simulink model "dds_cwg_32k_fft_only_wb"  (R2018a) (continued)
== system dds_cwg_32k_fft_only_wb == (continued)
      mask init:
      | simple_bram_vacc_init(gcb, ...
      |     'vec_len', vec_len, ...
      |     'arith_type', arith_type, ...
      |     'n_bits', n_bits, ...
      |     'bin_pt', bin_pt);
      mask TypeOptions arith_type: Signed | Unsigned
  simple_bram_vacc3 : Subsystem  Commented=on  AncestorBlock=casper_library_accumulators/simple_bram_vacc  mask(simple_bram_vacc){vec_len=2^13, arith_type=Signed, n_bits=32, bin_pt=7}
      mask prompts: vec_len "Vector length:"; arith_type "Output type:"; n_bits "Bit width (output):"; bin_pt "Binary point (output):"
      mask help: This simple vector accumulator is based on a shift register. It outputs its previous accumulated vector after a new_acc pulse is received.
      mask init:
      | simple_bram_vacc_init(gcb, ...
      |     'vec_len', vec_len, ...
      |     'arith_type', arith_type, ...
      |     'n_bits', n_bits, ...
      |     'bin_pt', bin_pt);
      mask TypeOptions arith_type: Signed | Unsigned
  simple_bram_vacc8 : Subsystem  Commented=on  AncestorBlock=casper_library_accumulators/simple_bram_vacc  mask(simple_bram_vacc){vec_len=2^13, arith_type=Signed, n_bits=32, bin_pt=7}
      mask prompts: vec_len "Vector length:"; arith_type "Output type:"; n_bits "Bit width (output):"; bin_pt "Binary point (output):"
      mask help: This simple vector accumulator is based on a shift register. It outputs its previous accumulated vector after a new_acc pulse is received.
      mask init:
      | simple_bram_vacc_init(gcb, ...
      |     'vec_len', vec_len, ...
      |     'arith_type', arith_type, ...
      |     'n_bits', n_bits, ...
      |     'bin_pt', bin_pt);
      mask TypeOptions arith_type: Signed | Unsigned
  snap_adc : Subsystem  Tag=casper:bitsnap  AncestorBlock=casper_library_scopes/bitfield_snapshot  mask(bitsnap){io_names=[p0_d0 p0_d1 p0_d2 p0_d3 p0_d4 p0_d5 p0_d6 p0_d7], io_widths=10, io_bps=9, io_types=1, show_format=on, snap_storage=bram, snap_dram_dimm=2, snap_dram_clock=250, snap_nsamples=10, snap_data_width=128, snap_offset=off, snap_circap=off, snap_value=off, snap_ext_arm=off, snap_ext_circ=off, snap_provide_outputs=on, snap_use_dsp48=on, snap_delay=2, extra_names=timestamp, extra_widths=32, extra_bps=0, extra_types=0}
      mask prompts: io_names "Names [msb...lsb]"; io_widths "Bitwidths [msb...lsb]"; io_bps "Binary pts [msb...lsb]"; io_types "Data types [msb...lsb], ufix=0, fix=1, bool=2"; show_format "Print format string?"; snap_storage "Storage medium"; snap_dram_dimm "DRAM dimm"; snap_dram_clock "DRAM clock rate"; snap_nsamples "Number of Samples (2^?)"; snap_data_width "Data width"; snap_offset "Start delay support"; snap_circap "Circular capture support"; snap_value "Extra value capture support"; snap_ext_arm "External arm support"; snap_ext_circ "External circular capture support"; snap_provide_outputs "Provide outputs"; snap_use_dsp48 "Use DSP48s to implement counters"; snap_delay "Pre-snapshot delay"; extra_names "Names [msb...lsb]"; extra_widths "Widths [msb...lsb]"; extra_bps "Binary pts [msb...lsb]"; extra_types "Data types [msb...lsb], ufix=0, fix=1, bool=2"
      mask help:
      | A wrapped snapshot block. Allows specification of inputs to a snapblock word so the toolflow can automatically set up the scope.
      | See documentation for snapshot for explanation of parameters.
      mask init:
      | bitsnap_init(gcb);
      settings: AttributesFormatString=|
      | snap(8):f10.9,f10.9,f10.9,f10.9,f10.9,f10.9,f10.9,f10.9 = 80 bits\n128 wide, 1024 deep
      | debugID: 20968299
      mask TypeOptions snap_storage: bram | dram
      mask Callback snap_storage: bitsnap_callback();
      mask TypeOptions snap_dram_dimm: 1 | 2 | 3 | 4
      mask TypeOptions snap_dram_clock: 200 | 250 | 266 | 300 | 333
      mask TypeOptions snap_data_width: 8 | 16 | 32 | 64 | 128
      mask Callback snap_value: bitsnap_callback();
      mask Callback snap_ext_arm: bitsnap_callback();
      mask Callback snap_ext_circ: bitsnap_callback();
      mask Callback snap_provide_outputs: bitsnap_callback();
      mask DialogControl ParameterTabVar0 Prompt: Inputs
      mask DialogControl ParameterTabVar01 Prompt: Snapshot
      mask DialogControl ParameterTabVar012 Prompt: Extra Value
  snap_quant0 : Subsystem  Tag=casper:bitsnap  Commented=on  AncestorBlock=casper_library_scopes/bitfield_snapshot  mask(bitsnap){io_names=[real0 imag0 real1 imag1 real2 imag2 real3 imag3], io_widths=8, io_bps=7, io_types=1, show_format=on, snap_storage=bram, snap_dram_dimm=2, snap_dram_clock=250, snap_nsamples=13, snap_data_width=64, snap_offset=on, snap_circap=off, snap_value=off, snap_ext_arm=off, snap_ext_circ=off, snap_provide_outputs=on, snap_use_dsp48=on, snap_delay=0, extra_names=[c d], extra_widths=[30 2], extra_bps=[0 0], extra_types=0}
      mask prompts: io_names "Names [msb...lsb]"; io_widths "Bitwidths [msb...lsb]"; io_bps "Binary pts [msb...lsb]"; io_types "Data types [msb...lsb], ufix=0, fix=1, bool=2"; show_format "Print format string?"; snap_storage "Storage medium"; snap_dram_dimm "DRAM dimm"; snap_dram_clock "DRAM clock rate"; snap_nsamples "Number of Samples (2^?)"; snap_data_width "Data width"; snap_offset "Start delay support"; snap_circap "Circular capture support"; snap_value "Extra value capture support"; snap_ext_arm "External arm support"; snap_ext_circ "External circular capture support"; snap_provide_outputs "Provide outputs"; snap_use_dsp48 "Use DSP48s to implement counters"; snap_delay "Pre-snapshot delay"; extra_names "Names [msb...lsb]"; extra_widths "Widths [msb...lsb]"; extra_bps "Binary pts [msb...lsb]"; extra_types "Data types [msb...lsb], ufix=0, fix=1, bool=2"
      mask help:
      | A wrapped snapshot block. Allows specification of inputs to a snapblock word so the toolflow can automatically set up the scope.
      | See documentation for snapshot for explanation of parameters.
      mask init:
      | bitsnap_init(gcb);
      settings: AttributesFormatString=|
      | snap(8):f8.7,f8.7,f8.7,f8.7,f8.7,f8.7,f8.7,f8.7 = 64 bits\n64 wide, 8192 deep
      | debugID: 82310566
      mask TypeOptions snap_storage: bram | dram
      mask Callback snap_storage: bitsnap_callback();
      mask TypeOptions snap_dram_dimm: 1 | 2 | 3 | 4
      mask TypeOptions snap_dram_clock: 200 | 250 | 266 | 300 | 333
      mask TypeOptions snap_data_width: 8 | 16 | 32 | 64 | 128
      mask Callback snap_value: bitsnap_callback();
      mask Callback snap_ext_arm: bitsnap_callback();
      mask Callback snap_ext_circ: bitsnap_callback();
      mask Callback snap_provide_outputs: bitsnap_callback();
      mask DialogControl ParameterTabVar0 Prompt: Inputs
      mask DialogControl ParameterTabVar01 Prompt: Snapshot
      mask DialogControl ParameterTabVar012 Prompt: Extra Value
  spect_cnt1 : xbsIndex_r4/Counter  Commented=on  cnt_type=Free Running  cnt_to=Inf  operation=Up  start_count=0  cnt_by_val=1  arith_type=Unsigned  n_bits=32  bin_pt=0  load_pin=off  rst=on  en=on  explicit_period=on  period=1  use_behavioral_HDL=off  implementation=Fabric  use_rpm=off  dbl_ovrd=off  block_type=counter
  spect_cnt2 : xbsIndex_r4/Counter  Commented=on  cnt_type=Free Running  cnt_to=Inf  operation=Up  start_count=0  cnt_by_val=1  arith_type=Unsigned  n_bits=32  bin_pt=0  load_pin=off  rst=on  en=on  explicit_period=on  period=1  use_behavioral_HDL=off  implementation=Fabric  use_rpm=off  dbl_ovrd=off  block_type=counter
  spect_cnt3 : xbsIndex_r4/Counter  Commented=on  cnt_type=Free Running  cnt_to=Inf  operation=Up  start_count=0  cnt_by_val=1  arith_type=Unsigned  n_bits=32  bin_pt=0  load_pin=off  rst=on  en=on  explicit_period=on  period=1  use_behavioral_HDL=off  implementation=Fabric  use_rpm=off  dbl_ovrd=off  block_type=counter
  ss_fft : Subsystem  Tag=casper:bitsnap  AncestorBlock=casper_library_scopes/bitfield_snapshot  mask(bitsnap){io_names=[r0 i0 r1 i1 r2 i2 r3 i3], io_widths=[10 10 10 10 10 10 10 10], io_bps=[9 9 9 9 9 9 9 9], io_types=[1 1 1 1 1 1 1 1], show_format=on, snap_storage=bram, snap_dram_dimm=2, snap_dram_clock=250, snap_nsamples=13, snap_data_width=128, snap_offset=off, snap_circap=off, snap_value=off, snap_ext_arm=off, snap_ext_circ=off, snap_provide_outputs=on, snap_use_dsp48=on, snap_delay=2, extra_names=[c d], extra_widths=[30 2], extra_bps=[0 0], extra_types=[0 0]}
      mask prompts: io_names "Names [msb...lsb]"; io_widths "Bitwidths [msb...lsb]"; io_bps "Binary pts [msb...lsb]"; io_types "Data types [msb...lsb], ufix=0, fix=1, bool=2"; show_format "Print format string?"; snap_storage "Storage medium"; snap_dram_dimm "DRAM dimm"; snap_dram_clock "DRAM clock rate"; snap_nsamples "Number of Samples (2^?)"; snap_data_width "Data width"; snap_offset "Start delay support"; snap_circap "Circular capture support"; snap_value "Extra value capture support"; snap_ext_arm "External arm support"; snap_ext_circ "External circular capture support"; snap_provide_outputs "Provide outputs"; snap_use_dsp48 "Use DSP48s to implement counters"; snap_delay "Pre-snapshot delay"; extra_names "Names [msb...lsb]"; extra_widths "Widths [msb...lsb]"; extra_bps "Binary pts [msb...lsb]"; extra_types "Data types [msb...lsb], ufix=0, fix=1, bool=2"
      mask help:
      | A wrapped snapshot block. Allows specification of inputs to a snapblock word so the toolflow can automatically set up the scope.
      | See documentation for snapshot for explanation of parameters.
      mask init:
      | bitsnap_init(gcb);
      settings: AttributesFormatString=|
      | snap(8):f10.9,f10.9,f10.9,f10.9,f10.9,f10.9,f10.9,f10.9 = 80 bits\n128 wide, 8192 deep
      | debugID: 107946561
      mask TypeOptions snap_storage: bram | dram
      mask Callback snap_storage: bitsnap_callback();
      mask TypeOptions snap_dram_dimm: 1 | 2 | 3 | 4
      mask TypeOptions snap_dram_clock: 200 | 250 | 266 | 300 | 333
      mask TypeOptions snap_data_width: 8 | 16 | 32 | 64 | 128
      mask Callback snap_value: bitsnap_callback();
      mask Callback snap_ext_arm: bitsnap_callback();
      mask Callback snap_ext_circ: bitsnap_callback();
      mask Callback snap_provide_outputs: bitsnap_callback();
      mask DialogControl ParameterTabVar0 Prompt: Inputs
      mask DialogControl ParameterTabVar01 Prompt: Snapshot
      mask DialogControl ParameterTabVar012 Prompt: Extra Value
  ss_quant : Subsystem  Tag=casper:bitsnap  Commented=on  AncestorBlock=casper_library_scopes/bitfield_snapshot  mask(bitsnap){io_names=[r0 i0 r1 i1 r2 i2 r3 i3], io_widths=[8 8 8 8 8 8 8 8], io_bps=[7 7 7 7 7 7 7 7], io_types=[1 1 1 1 1 1 1 1], show_format=on, snap_storage=bram, snap_dram_dimm=2, snap_dram_clock=250, snap_nsamples=13, snap_data_width=64, snap_offset=off, snap_circap=off, snap_value=off, snap_ext_arm=off, snap_ext_circ=off, snap_provide_outputs=on, snap_use_dsp48=on, snap_delay=2, extra_names=[c d], extra_widths=[30 2], extra_bps=[0 0], extra_types=[0 0]}
      mask prompts: io_names "Names [msb...lsb]"; io_widths "Bitwidths [msb...lsb]"; io_bps "Binary pts [msb...lsb]"; io_types "Data types [msb...lsb], ufix=0, fix=1, bool=2"; show_format "Print format string?"; snap_storage "Storage medium"; snap_dram_dimm "DRAM dimm"; snap_dram_clock "DRAM clock rate"; snap_nsamples "Number of Samples (2^?)"; snap_data_width "Data width"; snap_offset "Start delay support"; snap_circap "Circular capture support"; snap_value "Extra value capture support"; snap_ext_arm "External arm support"; snap_ext_circ "External circular capture support"; snap_provide_outputs "Provide outputs"; snap_use_dsp48 "Use DSP48s to implement counters"; snap_delay "Pre-snapshot delay"; extra_names "Names [msb...lsb]"; extra_widths "Widths [msb...lsb]"; extra_bps "Binary pts [msb...lsb]"; extra_types "Data types [msb...lsb], ufix=0, fix=1, bool=2"
      mask help:
      | A wrapped snapshot block. Allows specification of inputs to a snapblock word so the toolflow can automatically set up the scope.
      | See documentation for snapshot for explanation of parameters.
      mask init:
      | bitsnap_init(gcb);
      settings: AttributesFormatString=|
      | snap(8):f8.7,f8.7,f8.7,f8.7,f8.7,f8.7,f8.7,f8.7 = 64 bits\n64 wide, 8192 deep
      | debugID: 40187090
      mask TypeOptions snap_storage: bram | dram
      mask Callback snap_storage: bitsnap_callback();
      mask TypeOptions snap_dram_dimm: 1 | 2 | 3 | 4
      mask TypeOptions snap_dram_clock: 200 | 250 | 266 | 300 | 333
      mask TypeOptions snap_data_width: 8 | 16 | 32 | 64 | 128
      mask Callback snap_value: bitsnap_callback();
      mask Callback snap_ext_arm: bitsnap_callback();
      mask Callback snap_ext_circ: bitsnap_callback();
      mask Callback snap_provide_outputs: bitsnap_callback();
      mask DialogControl ParameterTabVar0 Prompt: Inputs
      mask DialogControl ParameterTabVar01 Prompt: Snapshot
      mask DialogControl ParameterTabVar012 Prompt: Extra Value
  ss_vacc0 : Subsystem  Tag=casper:bitsnap  Commented=on  AncestorBlock=casper_library_scopes/bitfield_snapshot  mask(bitsnap){io_names=[data], io_widths=[32], io_bps=[7], io_types=[1], show_format=on, snap_storage=bram, snap_dram_dimm=2, snap_dram_clock=250, snap_nsamples=13, snap_data_width=32, snap_offset=off, snap_circap=off, snap_value=off, snap_ext_arm=off, snap_ext_circ=off, snap_provide_outputs=on, snap_use_dsp48=on, snap_delay=2, extra_names=[c d], extra_widths=[30 2], extra_bps=[0 0], extra_types=[0 0]}
      mask prompts: io_names "Names [msb...lsb]"; io_widths "Bitwidths [msb...lsb]"; io_bps "Binary pts [msb...lsb]"; io_types "Data types [msb...lsb], ufix=0, fix=1, bool=2"; show_format "Print format string?"; snap_storage "Storage medium"; snap_dram_dimm "DRAM dimm"; snap_dram_clock "DRAM clock rate"; snap_nsamples "Number of Samples (2^?)"; snap_data_width "Data width"; snap_offset "Start delay support"; snap_circap "Circular capture support"; snap_value "Extra value capture support"; snap_ext_arm "External arm support"; snap_ext_circ "External circular capture support"; snap_provide_outputs "Provide outputs"; snap_use_dsp48 "Use DSP48s to implement counters"; snap_delay "Pre-snapshot delay"; extra_names "Names [msb...lsb]"; extra_widths "Widths [msb...lsb]"; extra_bps "Binary pts [msb...lsb]"; extra_types "Data types [msb...lsb], ufix=0, fix=1, bool=2"
      mask help:
      | A wrapped snapshot block. Allows specification of inputs to a snapblock word so the toolflow can automatically set up the scope.
      | See documentation for snapshot for explanation of parameters.
      mask init:
      | bitsnap_init(gcb);
      settings: AttributesFormatString=|
      | snap(1):f32.7 = 32 bits\n32 wide, 8192 deep
      | debugID: 41826704
      mask TypeOptions snap_storage: bram | dram
      mask Callback snap_storage: bitsnap_callback();
      mask TypeOptions snap_dram_dimm: 1 | 2 | 3 | 4
      mask TypeOptions snap_dram_clock: 200 | 250 | 266 | 300 | 333
      mask TypeOptions snap_data_width: 8 | 16 | 32 | 64 | 128
      mask Callback snap_value: bitsnap_callback();
      mask Callback snap_ext_arm: bitsnap_callback();
      mask Callback snap_ext_circ: bitsnap_callback();
      mask Callback snap_provide_outputs: bitsnap_callback();
      mask DialogControl ParameterTabVar0 Prompt: Inputs
      mask DialogControl ParameterTabVar01 Prompt: Snapshot
      mask DialogControl ParameterTabVar012 Prompt: Extra Value
  ss_vacc1 : Subsystem  Tag=casper:bitsnap  Commented=on  AncestorBlock=casper_library_scopes/bitfield_snapshot  mask(bitsnap){io_names=[data], io_widths=[32], io_bps=[7], io_types=[1], show_format=on, snap_storage=bram, snap_dram_dimm=2, snap_dram_clock=250, snap_nsamples=13, snap_data_width=32, snap_offset=off, snap_circap=off, snap_value=off, snap_ext_arm=off, snap_ext_circ=off, snap_provide_outputs=on, snap_use_dsp48=on, snap_delay=2, extra_names=[c d], extra_widths=[30 2], extra_bps=[0 0], extra_types=[0 0]}
      mask prompts: io_names "Names [msb...lsb]"; io_widths "Bitwidths [msb...lsb]"; io_bps "Binary pts [msb...lsb]"; io_types "Data types [msb...lsb], ufix=0, fix=1, bool=2"; show_format "Print format string?"; snap_storage "Storage medium"; snap_dram_dimm "DRAM dimm"; snap_dram_clock "DRAM clock rate"; snap_nsamples "Number of Samples (2^?)"; snap_data_width "Data width"; snap_offset "Start delay support"; snap_circap "Circular capture support"; snap_value "Extra value capture support"; snap_ext_arm "External arm support"; snap_ext_circ "External circular capture support"; snap_provide_outputs "Provide outputs"; snap_use_dsp48 "Use DSP48s to implement counters"; snap_delay "Pre-snapshot delay"; extra_names "Names [msb...lsb]"; extra_widths "Widths [msb...lsb]"; extra_bps "Binary pts [msb...lsb]"; extra_types "Data types [msb...lsb], ufix=0, fix=1, bool=2"
      mask help:
      | A wrapped snapshot block. Allows specification of inputs to a snapblock word so the toolflow can automatically set up the scope.
      | See documentation for snapshot for explanation of parameters.
      mask init:
      | bitsnap_init(gcb);
      settings: AttributesFormatString=|
      | snap(1):f32.7 = 32 bits\n32 wide, 8192 deep
      | debugID: 41856495
      mask TypeOptions snap_storage: bram | dram
      mask Callback snap_storage: bitsnap_callback();
      mask TypeOptions snap_dram_dimm: 1 | 2 | 3 | 4
      mask TypeOptions snap_dram_clock: 200 | 250 | 266 | 300 | 333
      mask TypeOptions snap_data_width: 8 | 16 | 32 | 64 | 128
      mask Callback snap_value: bitsnap_callback();
      mask Callback snap_ext_arm: bitsnap_callback();
      mask Callback snap_ext_circ: bitsnap_callback();
      mask Callback snap_provide_outputs: bitsnap_callback();
      mask DialogControl ParameterTabVar0 Prompt: Inputs
      mask DialogControl ParameterTabVar01 Prompt: Snapshot
      mask DialogControl ParameterTabVar012 Prompt: Extra Value
  ss_vacc2 : Subsystem  Tag=casper:bitsnap  Commented=on  AncestorBlock=casper_library_scopes/bitfield_snapshot  mask(bitsnap){io_names=[data], io_widths=[32], io_bps=[7], io_types=[1], show_format=on, snap_storage=bram, snap_dram_dimm=2, snap_dram_clock=250, snap_nsamples=13, snap_data_width=32, snap_offset=off, snap_circap=off, snap_value=off, snap_ext_arm=off, snap_ext_circ=off, snap_provide_outputs=on, snap_use_dsp48=on, snap_delay=2, extra_names=[c d], extra_widths=[30 2], extra_bps=[0 0], extra_types=[0 0]}
      mask prompts: io_names "Names [msb...lsb]"; io_widths "Bitwidths [msb...lsb]"; io_bps "Binary pts [msb...lsb]"; io_types "Data types [msb...lsb], ufix=0, fix=1, bool=2"; show_format "Print format string?"; snap_storage "Storage medium"; snap_dram_dimm "DRAM dimm"; snap_dram_clock "DRAM clock rate"; snap_nsamples "Number of Samples (2^?)"; snap_data_width "Data width"; snap_offset "Start delay support"; snap_circap "Circular capture support"; snap_value "Extra value capture support"; snap_ext_arm "External arm support"; snap_ext_circ "External circular capture support"; snap_provide_outputs "Provide outputs"; snap_use_dsp48 "Use DSP48s to implement counters"; snap_delay "Pre-snapshot delay"; extra_names "Names [msb...lsb]"; extra_widths "Widths [msb...lsb]"; extra_bps "Binary pts [msb...lsb]"; extra_types "Data types [msb...lsb], ufix=0, fix=1, bool=2"
      mask help:
      | A wrapped snapshot block. Allows specification of inputs to a snapblock word so the toolflow can automatically set up the scope.
      | See documentation for snapshot for explanation of parameters.
      mask init:
      | bitsnap_init(gcb);
      settings: AttributesFormatString=|
      | snap(1):f32.7 = 32 bits\n32 wide, 8192 deep
      | debugID: 41886286
      mask TypeOptions snap_storage: bram | dram
      mask Callback snap_storage: bitsnap_callback();
      mask TypeOptions snap_dram_dimm: 1 | 2 | 3 | 4
      mask TypeOptions snap_dram_clock: 200 | 250 | 266 | 300 | 333
      mask TypeOptions snap_data_width: 8 | 16 | 32 | 64 | 128
      mask Callback snap_value: bitsnap_callback();
      mask Callback snap_ext_arm: bitsnap_callback();
      mask Callback snap_ext_circ: bitsnap_callback();
      mask Callback snap_provide_outputs: bitsnap_callback();
      mask DialogControl ParameterTabVar0 Prompt: Inputs
      mask DialogControl ParameterTabVar01 Prompt: Snapshot
      mask DialogControl ParameterTabVar012 Prompt: Extra Value
  ss_vacc3 : Subsystem  Tag=casper:bitsnap  Commented=on  AncestorBlock=casper_library_scopes/bitfield_snapshot  mask(bitsnap){io_names=[data], io_widths=[32], io_bps=[7], io_types=[1], show_format=on, snap_storage=bram, snap_dram_dimm=2, snap_dram_clock=250, snap_nsamples=13, snap_data_width=32, snap_offset=off, snap_circap=off, snap_value=off, snap_ext_arm=off, snap_ext_circ=off, snap_provide_outputs=on, snap_use_dsp48=on, snap_delay=2, extra_names=[c d], extra_widths=[30 2], extra_bps=[0 0], extra_types=[0 0]}
      mask prompts: io_names "Names [msb...lsb]"; io_widths "Bitwidths [msb...lsb]"; io_bps "Binary pts [msb...lsb]"; io_types "Data types [msb...lsb], ufix=0, fix=1, bool=2"; show_format "Print format string?"; snap_storage "Storage medium"; snap_dram_dimm "DRAM dimm"; snap_dram_clock "DRAM clock rate"; snap_nsamples "Number of Samples (2^?)"; snap_data_width "Data width"; snap_offset "Start delay support"; snap_circap "Circular capture support"; snap_value "Extra value capture support"; snap_ext_arm "External arm support"; snap_ext_circ "External circular capture support"; snap_provide_outputs "Provide outputs"; snap_use_dsp48 "Use DSP48s to implement counters"; snap_delay "Pre-snapshot delay"; extra_names "Names [msb...lsb]"; extra_widths "Widths [msb...lsb]"; extra_bps "Binary pts [msb...lsb]"; extra_types "Data types [msb...lsb], ufix=0, fix=1, bool=2"
      mask help:
      | A wrapped snapshot block. Allows specification of inputs to a snapblock word so the toolflow can automatically set up the scope.
      | See documentation for snapshot for explanation of parameters.
      mask init:
      | bitsnap_init(gcb);
      settings: AttributesFormatString=|
      | snap(1):f32.7 = 32 bits\n32 wide, 8192 deep
      | debugID: 41916077
      mask TypeOptions snap_storage: bram | dram
      mask Callback snap_storage: bitsnap_callback();
      mask TypeOptions snap_dram_dimm: 1 | 2 | 3 | 4
      mask TypeOptions snap_dram_clock: 200 | 250 | 266 | 300 | 333
      mask TypeOptions snap_data_width: 8 | 16 | 32 | 64 | 128
      mask Callback snap_value: bitsnap_callback();
      mask Callback snap_ext_arm: bitsnap_callback();
      mask Callback snap_ext_circ: bitsnap_callback();
      mask Callback snap_provide_outputs: bitsnap_callback();
      mask DialogControl ParameterTabVar0 Prompt: Inputs
      mask DialogControl ParameterTabVar01 Prompt: Snapshot
      mask DialogControl ParameterTabVar012 Prompt: Extra Value
  wng0 : Subsystem  mask{seed1=hex2dec('12345678'), seed2=hex2dec('87654321'), seed3=hex2dec('45612378'), seed4=hex2dec('85274163')}
      mask prompts: seed1 "Seed 1"; seed2 "Seed 2"; seed3 "Seed 3"; seed4 "Seed 4"
  tag scope: cnt_rst=global, force_gbe_txen=global, local_time_capture=global, local_time_src=global, man_sync=global, pfb_bypass=global, snap_reord_mux_we_sel=global, snap_stop_mux_sel=global, sys_rst=global, ts_tvg_en=global
  wires:
  Constant21/1 -> gbe0/8(rx_overrun_ack)
  Constant20/1 -> gbe0/7(rx_ack)
  [gbe_rst] -> pipeline99/1(d)
  [gbe_cnt_rst] -> pipeline31/1(d)
  pipeline31/1(q) -> gbe0/9(debug_rst)
  gbe0/13(rx_dest_ip) -> pipeline115/1(d)
  gbe0/14(rx_dest_port) -> pipeline116/1(d)
  [rx_dest_ip] -> pipeline/1(d)
  pipeline/1(q) -> rx_dest_ip/1(out_reg)
  gbe0/1(led_up) -> pipeline100/1(d)
  [forty_gbe_led_up] -> pipeline20/1(d)
  gbe0/2(led_rx) -> pipeline101/1(d)
  [forty_gbe_led_rx] -> pipeline22/1(d)
  gbe0/3(led_tx) -> pipeline102/1(d)
  [forty_gbe_led_tx] -> pipeline51/1(d)
  gbe0/4(tx_afull) -> pipeline103/1(d)
  gbe0/5(tx_overflow) -> pipeline104/1(d)
  gbe0/12(rx_overrun) -> pipeline114/1(d)
  pipeline20/1(q) -> gbe_status/1(out_led_up)
  pipeline22/1(q) -> gbe_status/2(out_led_rx)
  pipeline51/1(q) -> gbe_status/3(out_led_tx)
  gbe0/8(rx_source_ip) -> pipeline110/1(d)
  gbe0/9(rx_source_port) -> pipeline111/1(d)
  gbe0/6(rx_data) -> pipeline108/1(d)
  gbe0/10(rx_end_of_frame) -> pipeline112/1(d)
  gbe0/11(rx_bad_frame) -> pipeline113/1(d)
  [s256_d] -> pipeline98/1(d)
  [s256_ip] -> pipeline97/1(d)
  [s256_port] -> pipeline96/1(d)
  Logical3/1 -> gbe0/6(tx_end_of_frame)
  [s256_v] -> Logical2/2
  [s256_eof] -> Logical3/2
  Delay4/1 -> Logical2/1, Logical3/1
  [s256_eof] -> Delay4/2
  pipeline96/1(q) -> gbe0/5(tx_dest_port)
  pipeline97/1(q) -> gbe0/4(tx_dest_ip)
  pipeline98/1(q) -> gbe0/2(tx_data)
  pipeline99/1(q) -> gbe0/1(rst)
  Logical5/1 -> Delay4/1
  pipeline100/1(q) -> [forty_gbe_led_up]
  pipeline101/1(q) -> [forty_gbe_led_rx]
  pipeline102/1(q) -> [forty_gbe_led_tx]
  pipeline103/1(q) -> [forty_gbe_tx_full]
  pipeline104/1(q) -> [forty_gbe_tx_of]
  pipeline108/1(q) -> [forty_gbe_rx_data]
  pipeline110/1(q) -> [forty_gbe_rx_src_ip]
  pipeline111/1(q) -> [forty_gbe_rx_src_port]
  pipeline112/1(q) -> [forty_gbe_rx_eof]
  pipeline113/1(q) -> [forty_gbe_rx_bad]
  pipeline114/1(q) -> [forty_gbe_rx_overrun]
  pipeline115/1(q) -> [rx_dest_ip]
  pipeline116/1(q) -> [rx_dest_port]
  Logical2/1 -> gbe0/3(tx_valid)
  gbe0/7(rx_valid) -> pipeline62/1(d)
  pipeline62/1(q) -> [forty_gbe_rx_valid]
  [gbe_txen] -> Logical5/2
  [force_gbe_txen] -> Logical7/2
  Logical7/1 -> Logical5/1
  edge_detect7/1(out) -> pipeline24/1(d)
  control/7(in_cnt_rst) -> edge_detect5/1(in)
  edge_detect5/1(out) -> pipeline18/1(d)
  control/3(in_gbe_rst) -> pipeline25/1(d)
  control/1(in_sys_rst) -> pipeline23/1(d)
  pipeline24/1(q) -> [clr_status]
  pipeline25/1(q) -> [gbe_rst]
  pipeline26/1(q) -> [gbe_txen]
  pipeline17/1(q) -> [tvg_adc0_en]
  control/5(in_tvg_adc0) -> pipeline17/1(d)
  edge_detect6/1(out) -> pipeline38/1(d)
  pipeline38/1(q) -> [gbe_cnt_rst]
  control/8(in_gbe_cnt_rst) -> edge_detect6/1(in)
  control/4(in_gbe_txen) -> pipeline26/1(d)
  control/2(in_status_clr) -> edge_detect7/1(in)
  pipeline43/1(q) -> [adc_snap_arm]
  control/9(in_adc_snap_arm) -> pipeline43/1(d)
  control/10(in_adc_snap_trig_select) -> pipeline39/1(d)
  pipeline39/1(q) -> [adc_snap_trig_source]
  pipeline59/1(q) -> [ct_tvg_en]
  control/11(in_ct_tvg_en) -> pipeline59/1(d)
  pipeline52/1(q) -> [up_dbg_rst]
  control/12(in_unpack_debug_reset) -> pipeline52/1(d)
  control/13(in_cd_bypass) -> pipeline131/1(d)
  pipeline93/1(q) -> [tvg_adc1_en]
  control/6(in_tvg_adc1) -> pipeline93/1(d)
  control/14(in_gbe_stopmux) -> pipeline27/1(d)
  pipeline27/1(q) -> [gbe_stopmux]
  control/15(in_pfb_bypass) -> pipeline66/1(d)
  pipeline66/1(q) -> [pfb_bypass]
  control/16(in_ts_tvg_en) -> pipeline148/1(d)
  pipeline148/1(q) -> [ts_tvg_en]
  control/17(in_force_gbe_txen) -> pipeline21/1(d)
  pipeline21/1(q) -> [force_gbe_txen]
  control/18(in_snap_stop_mux_sel) -> pipeline127/1(d)
  control/19(in_snap_reord_mux_we_sel) -> pipeline128/1(d)
  pipeline127/1(q) -> [snap_stop_mux_sel]
  pipeline128/1(q) -> [snap_reord_mux_we_sel]
  control/20(in_local_time_source) -> pipeline16/1(d)
  edge_detect8/1(out) -> pipeline164/1(d)
  pipeline164/1(q) -> [local_time_capture]
  pipeline16/1(q) -> [local_time_src]
  control/21(in_local_time_capture) -> edge_detect8/1(in)
  control/22(in_time_diff_check_en) -> pipeline47/1(d)
  pipeline47/1(q) -> [time_diff_check_en]
  control/23(in_auto_rst_enable) -> pipeline132/1(d)
  pipeline132/1(q) -> [auto_rst_enable]
  pipeline155/1(q) -> [pipeline_stall]
  control/24(in_pipeline_stall) -> pipeline155/1(d)
  [board_in_fault] -> Inverter/1
  Inverter/1 -> Logical7/1
  Constant18/1 -> [board_in_fault]
  Constant22/1 -> [s256_d]
  Constant23/1 -> [s256_v]
  Constant24/1 -> [s256_eof]
  Constant25/1 -> [s256_port]
  Constant26/1 -> [s256_ip]
  fft_shift/1(in_fft_shift) -> pipeline4/1(d)
  pipeline4/1(q) -> Gateway Out66/1, [fft_shift]
  Delay3/1 -> bus_expand6/1(bus_in), quant0/2(din)
  quant0/3(dvalid) -> Goto_pfb_sync4/1
  pipeline2/1(q) -> eq0/1(addr)
  pipeline41/1(q) -> Counter4/2
  Delay52/1 -> Gateway Out70/1, quant0/3(en)
  Counter4/1 -> pipeline2/1(d)
  quant0/4(of) -> Goto_pfb_sync5/1
  Constant15/1 -> eq0/2(data_in)
  quant0/1(sync_out) -> Goto_pfb_sync1/1
  Constant27/1 -> eq0/3(we)
  Delay51/1 -> Gateway Out69/1, quant0/1(sync_in)
  quant0/2(dout) -> Goto_pfb_sync2/1
  pipeline42/1(q) -> Counter4/1
  Gateway Out24/1 -> To Workspace25/1
  bus_expand3/1(msb_out8) -> Gateway Out24/1
  Gateway Out23/1 -> To Workspace23/1
  bus_expand3/2(out7) -> Gateway Out23/1
  Gateway Out25/1 -> To Workspace24/1
  bus_expand3/3(out6) -> Gateway Out25/1
  Gateway Out26/1 -> To Workspace29/1
  bus_expand3/4(out5) -> Gateway Out26/1
  Gateway Out27/1 -> To Workspace26/1
  bus_expand3/5(out4) -> Gateway Out27/1
  Gateway Out28/1 -> To Workspace32/1
  bus_expand3/6(out3) -> Gateway Out28/1
  Gateway Out29/1 -> To Workspace27/1
  bus_expand3/7(out2) -> Gateway Out29/1
  Gateway Out32/1 -> To Workspace35/1
  bus_expand3/8(lsb_out1) -> Gateway Out32/1
  From22/1 -> Gateway Out22/1
  Gateway Out22/1 -> To Workspace22/1
  Gateway Out21/1 -> To Workspace17/1
  From15/1 -> Gateway Out21/1
  From26/1 -> bus_expand3/1(bus_in)
  Gateway Out35/1 -> To Workspace28/1
  From16/1 -> Gateway Out35/1
  Delay15/1 -> Delay17/1
  From52/1 -> Delay32/1
  Delay27/1 -> Logical/1, Delay24/1
  Constant37/1 -> acc_scale/1(sim_1)
  Constant39/1 -> acc_len/1(sim_1)
  imag_square3/1 -> AddSub6/2
  Delay9/1 -> scale2/2(imag)
  real_square/1 -> AddSub/1
  real_square3/1 -> AddSub6/1
  simple_bram_vacc2/2(valid) -> Terminator11/1
  simple_bram_vacc3/2(valid) -> Terminator12/1
  real_square2/1 -> AddSub5/1
  pipeline78/1(q) -> real_square/2, real_square/1
  simple_bram_vacc8/2(valid) -> Goto40/1
  pipeline80/1(q) -> real_square3/2, real_square3/1
  Logical/1 -> acc_cntrl2/3(rst)
  Delay32/1 -> Delay28/1
  pipeline56/1(q) -> simple_bram_vacc3/2(din)
  From60/1 -> simple_bram_vacc3/1(new_acc)
  pipeline85/1(q) -> Convert2/1
  pipeline64/1(q) -> imag_square2/1, imag_square2/2
  Gateway Out46/1 -> To Workspace45/1
  Gateway Out41/1 -> To Workspace49/1
  From58/1 -> simple_bram_vacc2/1(new_acc)
  Convert2/1 -> pipeline49/1(d), Gateway Out36/1
  Convert1/1 -> pipeline32/1(d), Gateway Out34/1
  pipeline30/1(q) -> simple_bram_vacc8/2(din)
  Gateway Out47/1 -> To Workspace46/1
  Convert4/1 -> pipeline30/1(d), Gateway Out33/1
  From31/1 -> simple_bram_vacc8/1(new_acc)
  Gateway Out49/1 -> To Workspace48/1
  imag_square2/1 -> AddSub5/2
  From63/1 -> Gateway Out46/1
  Convert3/1 -> pipeline56/1(d), Gateway Out44/1
  From38/1 -> scale2/3(scale)
  From30/1 -> acc_cntrl2/1(acc_len)
  scale2/1(real_out) -> pipeline63/1(d)
  Delay31/1 -> Delay11/1
  pipeline60/1(q) -> imag_square1/2, imag_square1/1
  pipeline49/1(q) -> simple_bram_vacc2/2(din)
  From37/1 -> scale1/3(scale)
  Delay10/1 -> Delay33/1
  From48/1 -> scale/3(scale)
  simple_bram_vacc2/1(dout) -> Goto43/1
  acc_cntrl2/1(new_acc) -> Goto34/1
  imag_square1/1 -> AddSub4/2
  pipeline13/1(q) -> Goto37/1
  pipeline82/1(q) -> Delay27/1
  pipeline61/1(q) -> real_square1/1, real_square1/2
  pipeline79/1(q) -> imag_square3/2, imag_square3/1
  Delay29/1 -> scale1/1(real)
  AddSub4/1 -> pipeline84/1(d)
  acc_len/1(in_reg) -> pipeline13/1(d)
  Delay11/1 -> scale1/2(imag)
  Gateway Out44/1 -> To Workspace43/1
  pipeline63/1(q) -> real_square2/2, real_square2/1
  AddSub5/1 -> pipeline85/1(d)
  From43/1 -> Gateway Out37/1
  acc_scale/1(in_reg) -> pipeline71/1(d)
  pipeline77/1(q) -> imag_square/1, imag_square/2
  scale/2(imag_out) -> pipeline77/1(d)
  Delay17/1 -> scale/1(real)
  scale/1(real_out) -> pipeline78/1(d)
  Delay22/1 -> scale3/1(real)
  pipeline86/1(q) -> Convert3/1
  Delay33/1 -> pipeline81/1(d)
  Gateway Out57/1 -> To Workspace52/1
  Delay30/1 -> Delay26/1
  AddSub/1 -> pipeline83/1(d)
  Delay24/1 -> acc_cntrl2/2(sync), Gateway Out72/1
  real_square1/1 -> AddSub4/1
  From39/1 -> scale3/3(scale)
  pipeline32/1(q) -> simple_bram_vacc1/2(din)
  scale3/1(real_out) -> pipeline80/1(d)
  pipeline81/1(q) -> Delay30/1
  pipeline71/1(q) -> Goto32/1
  From57/1 -> Delay23/1
  scale1/2(imag_out) -> pipeline60/1(d)
  From32/1 -> Logical/2
  pipeline83/1(q) -> Convert5/1, Gateway Out48/1, Convert4/1
  scale2/2(imag_out) -> pipeline64/1(d)
  From55/1 -> Delay19/1
  Delay18/1 -> scale2/1(real)
  imag_square/1 -> AddSub/2
  From65/1 -> Gateway Out41/1
  scale1/1(real_out) -> pipeline61/1(d)
  From56/1 -> Delay20/1
  Delay26/1 -> pipeline82/1(d)
  simple_bram_vacc8/1(dout) -> Goto39/1
  Gateway Out48/1 -> To Workspace47/1
  Delay5/1 -> Delay29/1
  Delay14/1 -> Delay10/1
  Delay28/1 -> scale/2(imag)
  From59/1 -> simple_bram_vacc1/1(new_acc)
  Delay21/1 -> scale3/2(imag)
  pipeline84/1(q) -> Convert1/1
  simple_bram_vacc3/1(dout) -> Goto44/1
  Delay23/1 -> Delay21/1
  From50/1 -> Delay15/1
  From62/1 -> Gateway Out47/1
  scale3/2(imag_out) -> pipeline79/1(d)
  simple_bram_vacc1/1(dout) -> Goto42/1
  From66/1 -> Gateway Out57/1
  From33/1 -> Delay5/1
  AddSub6/1 -> pipeline86/1(d)
  From34/1 -> Delay14/1
  Delay19/1 -> Delay9/1
  From64/1 -> Gateway Out49/1
  Delay8/1 -> Delay18/1
  simple_bram_vacc1/2(valid) -> Terminator9/1
  Delay20/1 -> Delay22/1
  From53/1 -> Delay31/1
  From54/1 -> Delay8/1
  From67/1 -> bus_expand4/1(bus_in)
  bus_expand4/1(msb_out8) -> Goto35/1
  bus_expand4/2(out7) -> Goto45/1
  bus_expand4/3(out6) -> Goto46/1
  bus_expand4/4(out5) -> Goto47/1
  bus_expand4/5(out4) -> Goto48/1
  bus_expand4/6(out3) -> Goto49/1
  bus_expand4/7(out2) -> Goto50/1
  bus_expand4/8(lsb_out1) -> Goto51/1
  bus_expand5/6(out3) -> snap_quant0/6(in_imag2)
  bus_expand5/5(out4) -> snap_quant0/5(in_real2)
  bus_expand5/2(out7) -> snap_quant0/2(in_imag0)
  bus_expand5/3(out6) -> snap_quant0/3(in_real1)
  bus_expand5/8(lsb_out1) -> snap_quant0/8(in_imag3)
  bus_expand5/7(out2) -> snap_quant0/7(in_real3)
  bus_expand5/1(msb_out8) -> snap_quant0/1(in_real0)
  bus_expand5/4(out5) -> snap_quant0/4(in_imag1)
  pipeline15/1(q) -> bus_expand5/1(bus_in)
  pipeline90/1(q) -> snap_quant0/9(we)
  pipeline91/1(q) -> snap_quant0/10(trig)
  Gateway Out33/1 -> To Workspace33/1
  Gateway Out34/1 -> To Workspace34/1
  Gateway Out36/1 -> To Workspace36/1
  Gateway Out37/1 -> To Workspace37/1
  eq/1(in_eq) -> pipeline92/1(d)
  pipeline92/1(q) -> Goto52/1
  Constant38/1 -> eq/1(sim_1)
  From71/1 -> eq_const_bus/1(In1)
  eq_const_bus/1(Out1) -> quant0/4(coeffs)
  pipeline201/1(q) -> Delay145/1
  Delay146/1 -> pipeline201/1(d)
  Delay145/1 -> Delay143/1
  Delay142/1 -> Delay141/1
  From308/1 -> Delay142/1
  Delay143/1 -> pipeline202/1(d)
  Delay141/1 -> Delay146/1
  pipeline202/1(q) -> Delay144/1
  pipeline65/1(q) -> simple_bram_vacc8/3(dv)
  Delay144/1 -> Goto54/1, acc_cntrl2/4(dv)
  From115/1 -> Gateway Out71/1, pipeline65/1(d)
  Gateway Out71/1 -> To Workspace58/1
  Gateway Out72/1 -> To Workspace72/1
  [pfbin_sync] -> fft_only/2(sync_in)
  [pfbin_d0] -> fft_only/4(din1), fft_only/3(din0)
  [pfbin_dv] -> fft_only/5(en)
  [fft_shift] -> fft_only/1(shift)
  pipeline8/1(q) -> simple_bram_vacc1/3(dv)
  pipeline14/1(q) -> simple_bram_vacc2/3(dv)
  From78/1 -> pipeline8/1(d)
  From80/1 -> pipeline14/1(d)
  pipeline106/1(q) -> simple_bram_vacc3/3(dv)
  From81/1 -> pipeline106/1(d)
  Reinterpret3/1 -> Delay120/1
  Concat8/1 -> pipeline143/1(d)
  Constant57/1 -> delay_whole1/1(sim_1)
  Constant47/1 -> delay1_tl_control0/2(sim_2)
  delay0_tl_control0/2(in_load_immediate) -> pipeline135/1(d)
  phase_rate0/1(in_delta) -> Reinterpret4/1
  Constant58/1 -> phase1/1(sim_1)
  delay0_tl_control1/1(in_load_time_lsw) -> Concat6/2
  Reinterpret5/1 -> Delay44/1
  Delay115/1 -> pipeline170/1(d)
  Delay44/1 -> Concat3/1
  Constant49/1 -> Delay13/1
  pipeline173/1(q) -> Concat14/1
  pipeline169/1(q) -> Concat22/1
  delay_frac1/1(in_initial) -> Reinterpret2/1
  pipeline176/1(q) -> Concat14/4
  Delay119/1 -> pipeline174/1(d)
  Constant44/1 -> delay0_tl_control1/1(sim_1)
  Concat14/1 -> [ld_val1]
  delay1_tl_control0/1(in_arm) -> pipeline137/1(d)
  Reinterpret6/1 -> Delay119/1
  Reinterpret4/1 -> Delay121/1
  Constant41/1 -> delta_delay1/1(sim_1)
  Constant48/1 -> delay1_tl_control1/1(sim_1)
  Constant42/1 -> delay0_tl_control0/3(sim_3)
  Concat11/1 -> pipeline169/1(d)
  Delay118/1 -> Concat11/1
  pipeline134/1(q) -> [tl_delay0_arm]
  pipeline174/1(q) -> Concat14/2
  pipeline135/1(q) -> [tl_delay0_load_immediate]
  delay1_tl_control1/1(in_load_time_lsw) -> Concat8/2
  phase1/1(in_initial) -> Reinterpret9/1
  pipeline136/1(q) -> [tl_delay0_load_time]
  delay_frac0/1(in_initial) -> Reinterpret18/1
  Delay114/1 -> Concat3/2
  delay1_tl_control0/3(in_load_time_msw) -> Concat8/1
  Constant40/1 -> phase0/1(sim_1)
  Constant76/1 -> delay_frac0/1(sim_1)
  Constant43/1 -> delay0_tl_control0/2(sim_2)
  Constant75/1 -> phase_rate0/1(sim_1)
  Concat3/1 -> pipeline173/1(d)
  delta_delay1/1(in_initial) -> Reinterpret6/1
  pipeline172/1(q) -> Concat22/4
  delay_whole1/1(in_initial) -> Reinterpret5/1
  delay0_tl_control0/1(in_arm) -> pipeline134/1(d)
  pipeline171/1(q) -> Concat22/3
  phase_rate1/1(in_delta) -> Reinterpret12/1
  delta_delay0/1(in_initial) -> Reinterpret20/1
  Reinterpret20/1 -> Delay115/1
  Delay124/1 -> pipeline175/1(d)
  Reinterpret12/1 -> Delay125/1
  pipeline170/1(q) -> Concat22/2
  Delay125/1 -> pipeline176/1(d)
  Reinterpret2/1 -> Delay114/1
  Delay120/1 -> pipeline171/1(d)
  Reinterpret19/1 -> Delay118/1
  pipeline138/1(q) -> [tl_delay1_load_immediate]
  Reinterpret18/1 -> Delay42/1
  Concat22/1 -> [ld_val0]
  Delay12/1 -> delay0_tl_control0/1(sim_1)
  phase0/1(in_initial) -> Reinterpret3/1
  pipeline143/1(q) -> [tl_delay1_load_time]
  Concat6/1 -> pipeline136/1(d)
  Delay42/1 -> Concat11/2
  pipeline137/1(q) -> [tl_delay1_arm]
  Constant56/1 -> phase_rate1/1(sim_1)
  Delay121/1 -> pipeline172/1(d)
  Constant78/1 -> delta_delay0/1(sim_1)
  delay_whole0/1(in_initial) -> Reinterpret19/1
  pipeline175/1(q) -> Concat14/3
  delay0_tl_control0/3(in_load_time_msw) -> Concat6/1
  Constant50/1 -> delay_frac1/1(sim_1)
  Delay13/1 -> delay1_tl_control0/1(sim_1)
  Reinterpret9/1 -> Delay124/1
  delay1_tl_control0/2(in_load_immediate) -> pipeline138/1(d)
  Constant45/1 -> Delay12/1
  Constant46/1 -> delay1_tl_control0/3(sim_3)
  Constant31/1 -> delay_whole0/1(sim_1)
  pipeline131/1(q) -> [cd_bypass]
  man_sync/1(in_sync) -> Delay36/1
  pipeline18/1(q) -> [cnt_rst]
  From_pfb_of/1 -> Delay51/1, pipeline42/1(d)
  From_pfb_of2/1 -> Delay3/1
  From_pfb_of1/1 -> Delay52/1, pipeline41/1(d)
  From207/1 -> Goto118/1
  From200/1 -> Goto111/1
  From206/1 -> Goto117/1
  edge_detect11/1(out) -> [man_sync]
  From69/1 -> generate_pkt_sync1/1(sync)
  From70/1 -> generate_pkt_sync1/2(valid)
  From68/1 -> generate_pkt_sync1/3(data)
  generate_pkt_sync1/4(packet_sync) -> pipeline91/1(d)
  generate_pkt_sync1/3(out_data) -> pipeline15/1(d)
  generate_pkt_sync1/2(out_valid) -> pipeline90/1(d)
  [d80_adc0] -> [pfbin_d0]
  [sync80] -> [pfbin_sync]
  [valid80] -> [pfbin_dv]
  fft_only/1(sync_out) -> Delay43/1
  Gateway Out18/1 -> To Workspace21/1
  bus_expand1/1(msb_out8) -> Gateway Out12/1
  Gateway Out16/1 -> To Workspace19/1
  Gateway Out17/1 -> To Workspace16/1
  Gateway Out13/1 -> To Workspace13/1
  Gateway Out11/1 -> To Workspace12/1
  Gateway Out10/1 -> To Workspace10/1
  Gateway Out12/1 -> To Workspace14/1
  bus_expand1/8(lsb_out1) -> Gateway Out18/1
  [pfb_dv] -> Gateway Out10/1
  bus_expand1/2(out7) -> Gateway Out11/1
  bus_expand1/7(out2) -> Gateway Out17/1
  Gateway Out1/1 -> To Workspace1/1
  Gateway Out15/1 -> To Workspace15/1
  Gateway Out14/1 -> To Workspace18/1
  [pfb_sync] -> Gateway Out1/1
  [pfb_d144_0] -> bus_expand9/1(bus_in), bus_expand1/1(bus_in)
  bus_expand1/5(out4) -> Gateway Out15/1
  bus_expand1/6(out3) -> Gateway Out16/1
  bus_expand1/3(out6) -> Gateway Out13/1
  bus_expand1/4(out5) -> Gateway Out14/1
  Gateway Out59/1 -> To Workspace60/1
  Constant63/1 -> pkt_len/1(sim_1)
  Constant60/1 -> gap_sel/1(sim_1)
  Delay34/1 -> [cwg_dv], Gateway Out52/1
  edge_detect2/1(out) -> cwg/1(sync), Gateway Out30/1
  Constant52/1 -> White Gaussian Noise1/2
  Gateway Out54/1 -> To Workspace55/1
  Reinterpret7/1 -> Slice2/1
  AddSub7/1 -> Mux1/3
  Constant36/1 -> cwg_scale/1(sim_1)
  Relational3/1 -> Logical1/2
  [sys_rst] -> Register2/2
  Logical1/1 -> Gateway Out55/1, Register/2, Counter1/1
  [sys_rst] -> Logical1/1
  [sys_rst] -> cwg/4(rst)
  Gateway Out31/1 -> To Workspace31/1
  Gateway Out53/1 -> To Workspace54/1
  Delay2/1 -> Register2/1, Register2/3, Delay6/1
  pipeline11/1(q) -> Mux1/1
  Delay38/1 -> [cwg80]
  Mux1/1 -> Register/1
  pipeline1/1(q) -> Mux1/2, AddSub7/1
  cwg_scale/1(in_scale) -> Gateway Out53/1, cwg/5(scale)
  gap_sel/1(in_sel) -> pipeline11/1(d)
  Gateway Out40/1 -> To Workspace40/1
  [pkt_len] -> Relational1/2
  Register/1 -> AddSub8/1, Gateway Out56/1
  Delay6/1 -> Gateway Out31/1, cwg/3(dv)
  AddSub8/1 -> Gateway Out58/1, Relational3/2
  Slice1/1 -> AddSub7/2
  freq_cwg_input/1(in_frequency) -> cwg/2(freq), Gateway Out54/1
  pkt_len_sel/1(in_sel) -> pipeline19/1(d)
  Constant68/1 -> [osc_temp_dv]
  Constant62/1 -> pkt_len_sel/1(sim_1)
  Gateway Out30/1 -> To Workspace30/1
  Slice2/1 -> AddSub1/2
  AddSub1/1 -> Mux2/3
  Mux2/1 -> Gateway Out59/1, [pkt_len]
  Constant69/1 -> gap_offset/1(sim_1)
  White Gaussian Noise1/1 -> Reinterpret1/1
  pkt_len/1(in_len) -> pipeline9/1(d)
  Counter1/1 -> Relational3/1, Relational1/1
  cwg/3(dv_out) -> Delay34/1
  cwg/2(data_out) -> Delay38/1
  [osc_temp_dv] -> Delay2/1
  gap_offset/1(in_offset) -> pipeline1/1(d)
  cwg/1(sync_out) -> Gateway Out40/1, [cwg_sync]
  Constant70/1 -> AddSub8/2
  Gateway Out52/1 -> To Workspace53/1
  Gateway Out55/1 -> To Workspace56/1
  Gateway Out58/1 -> To Workspace59/1
  pipeline19/1(q) -> Mux2/1
  Gateway Out56/1 -> To Workspace57/1
  White Gaussian Noise1/2 -> Reinterpret7/1
  Reinterpret1/1 -> Slice1/1
  pipeline9/1(q) -> AddSub1/1, Mux2/2
  Constant51/1 -> White Gaussian Noise1/1
  bus_expand2/1(msb_out8) -> Gateway Out64/1
  bus_expand2/3(out6) -> Gateway Out65/1
  [d80_adc0] -> bus_expand2/1(bus_in)
  Gateway Out66/1 -> To Workspace68/1
  Gateway Out68/1 -> To Workspace69/1
  [pfb_of] -> Gateway Out68/1
  Gateway Out69/1 -> To Workspace70/1
  Gateway Out70/1 -> To Workspace71/1
  Gateway Out74/1 -> To Workspace74/1
  Gateway Out75/1 -> To Workspace75/1
  bus_expand6/1(msb_out8) -> Gateway Out75/1
  bus_expand6/2(out7) -> Gateway Out74/1
  Gateway Out78/1 -> To Workspace78/1
  Gateway Out79/1 -> To Workspace79/1
  bus_expand6/4(out5) -> Gateway Out78/1
  bus_expand6/3(out6) -> Gateway Out79/1
  Gateway Out81/1 -> To Workspace81/1
  Gateway Out80/1 -> To Workspace80/1
  bus_expand6/6(out3) -> Gateway Out80/1
  bus_expand6/5(out4) -> Gateway Out81/1
  Gateway Out82/1 -> To Workspace82/1
  Gateway Out83/1 -> To Workspace83/1
  bus_expand6/8(lsb_out1) -> Gateway Out82/1
  bus_expand6/7(out2) -> Gateway Out83/1
  Gateway Out76/1 -> To Workspace76/1
  Convert5/1 -> Gateway Out76/1
  From51/1 -> pipeline68/1(d)
  Gateway Out43/1 -> To Workspace41/1
  edge_detect1/1(out) -> ss_vacc3/3(trig)
  Register6/1 -> Inverter1/1
  From41/1 -> pipeline87/1(d)
  From90/1 -> pipeline3/1(d)
  From36/1 -> pipeline70/1(d)
  pipeline75/1(q) -> ss_vacc2/2(we)
  From21/1 -> Gateway Out89/1
  Gateway Out87/1 -> To Workspace87/1
  From40/1 -> pipeline6/1(d)
  pipeline72/1(q) -> ss_vacc1/2(we)
  pipeline87/1(q) -> ss_vacc3/1(in_data), Gateway Out50/1
  pipeline88/1(q) -> ss_vacc3/2(we)
  edge_detect10/1(out) -> Logical6/1
  From86/1 -> pipeline72/1(d)
  pipeline70/1(q) -> edge_detect4/1(in)
  ss_vacc0/1(arm_out) -> Terminator1/1
  Logical6/1 -> spect_cnt1/2
  pipeline69/1(q) -> Gateway Out7/1, ss_vacc0/2(we)
  From61/1 -> pipeline7/1(d)
  edge_detect4/1(out) -> Gateway Out6/1, ss_vacc0/3(trig)
  From84/1 -> pipeline69/1(d)
  From49/1 -> pipeline5/1(d)
  ss_vacc3/1(arm_out) -> Terminator8/1
  pipeline5/1(q) -> ss_vacc1/1(in_data), Gateway Out87/1
  From42/1 -> pipeline45/1(d)
  Gateway Out50/1 -> To Workspace42/1
  ss_vacc2/1(arm_out) -> Terminator7/1
  From35/1 -> spect_cnt1/1, Register6/2
  From45/1 -> pipeline88/1(d)
  From87/1 -> pipeline75/1(d)
  edge_detect9/1(out) -> ss_vacc2/3(trig)
  pipeline74/1(q) -> ss_vacc2/1(in_data), Gateway Out43/1
  From44/1 -> pipeline74/1(d)
  Gateway Out86/1 -> To Workspace86/1
  edge_detect3/1(out) -> ss_vacc1/3(trig)
  edge_detect14/1(out) -> Goto60/1
  pipeline68/1(q) -> ss_vacc0/1(in_data), Gateway Out86/1
  Gateway Out89/1 -> To Workspace89/1
  ss_vacc1/1(arm_out) -> Terminator6/1
  pipeline29/1(q) -> Relational4/2
  spect_cnt1/1 -> Relational4/1
  From85/1 -> pipeline29/1(d)
  pipeline45/1(q) -> edge_detect10/1(in)
  Inverter1/1 -> Delay39/1, Logical6/2
  Relational4/1 -> Logical4/1
  Logical4/1 -> Register6/3, Register6/1, edge_detect14/1(in)
  Delay/1 -> Logical4/2
  Delay39/1 -> Delay/1
  edge_detect15/1(out) -> ss_quant/10(trig)
  ss_quant/1(arm_out) -> Terminator2/1
  From4/1 -> pipeline76/1(d)
  From1/1 -> pipeline28/1(d)
  From7/1 -> pipeline94/1(d)
  From23/1 -> pipeline105/1(d)
  From89/1 -> pipeline109/1(d)
  From88/1 -> pipeline107/1(d)
  From8/1 -> pipeline95/1(d)
  From5/1 -> pipeline89/1(d)
  From92/1 -> pipeline117/1(d)
  pipeline28/1(q) -> ss_quant/1(in_r0)
  pipeline76/1(q) -> ss_quant/2(in_i0)
  pipeline89/1(q) -> ss_quant/3(in_r1)
  pipeline94/1(q) -> ss_quant/4(in_i1)
  pipeline95/1(q) -> ss_quant/5(in_r2)
  pipeline105/1(q) -> ss_quant/6(in_i2)
  pipeline107/1(q) -> ss_quant/7(in_r3)
  pipeline109/1(q) -> ss_quant/8(in_i3)
  pipeline117/1(q) -> ss_quant/9(we)
  From93/1 -> pipeline118/1(d)
  pipeline118/1(q) -> edge_detect15/1(in)
  From77/1 -> spect_cnt2/2, Goto4/1
  spect_cnt2/1 -> Delay40/1
  Convert/1 -> Goto36/1
  Convert6/1 -> Goto29/1
  Convert7/1 -> Goto11/1
  Convert8/1 -> Goto8/1
  Gateway Out2/1 -> To Workspace2/1
  From73/1 -> Gateway Out2/1
  From82/1 -> spect_cnt3/1, Logical8/2
  Delay7/1 -> acc_cntrl1/2(sync)
  acc_cntrl1/1(new_acc) -> Goto63/1
  From79/1 -> Gateway Out4/1, acc_cntrl1/1(acc_len)
  Logical8/1 -> acc_cntrl1/3(rst)
  From74/1 -> Delay7/1, Logical8/1, spect_cnt2/1, Goto3/1
  spect_cnt3/1 -> Goto6/1
  Slice/1 -> Relational/2
  Relational/1 -> spect_cnt3/2
  Constant/1 -> Relational/1
  Gateway Out3/1 -> To Workspace3/1
  From83/1 -> Gateway Out3/1
  Constant2/1 -> edge_detect12/1(in), Delay37/1
  Delay37/1 -> Goto64/1
  edge_detect12/1(out) -> Goto62/1
  Gateway Out4/1 -> To Workspace4/1
  From95/1 -> acc_cntrl1/4(dv)
  Delay40/1 -> Convert/1, Convert6/1, Convert7/1, Convert8/1, Slice/1
  Gateway Out5/1 -> To Workspace5/1
  From100/1 -> Gateway Out5/1
  Gateway Out6/1 -> To Workspace6/1
  Gateway Out7/1 -> To Workspace7/1
  Constant83/1 -> freq_cwg_input/1(sim_1)
  pipeline3/1(q) -> edge_detect1/1(in)
  pipeline6/1(q) -> edge_detect9/1(in)
  pipeline7/1(q) -> edge_detect3/1(in)
  bus_expand7/8(lsb_out1) -> snap_adc/8(in_p0_d7)
  bus_expand7/4(out5) -> snap_adc/4(in_p0_d3)
  bus_expand7/1(msb_out8) -> snap_adc/1(in_p0_d0)
  bus_expand7/3(out6) -> snap_adc/3(in_p0_d2)
  Delay16/1 -> bus_expand7/1(bus_in)
  [valid80] -> Delay25/1
  bus_expand7/2(out7) -> snap_adc/2(in_p0_d1)
  bus_expand7/7(out2) -> snap_adc/7(in_p0_d6)
  [d80_adc0] -> Delay16/1
  Delay25/1 -> snap_adc/9(we)
  bus_expand7/6(out3) -> snap_adc/6(in_p0_d5)
  bus_expand7/5(out4) -> snap_adc/5(in_p0_d4)
  [sync80] -> Delay35/1
  Delay35/1 -> snap_adc/10(trig)
  pipeline36/1(q) -> edge_detect13/1(in)
  [pfb_sync] -> pipeline36/1(d)
  pipeline35/1(q) -> ss_fft/9(we)
  pipeline34/1(q) -> ss_fft/8(in_i3)
  pipeline33/1(q) -> ss_fft/7(in_r3)
  pipeline12/1(q) -> ss_fft/6(in_i2)
  pipeline48/1(q) -> ss_fft/5(in_r2)
  pipeline46/1(q) -> ss_fft/4(in_i1)
  pipeline44/1(q) -> ss_fft/3(in_r1)
  pipeline40/1(q) -> ss_fft/2(in_i0)
  pipeline37/1(q) -> ss_fft/1(in_r0)
  [pfb_dv] -> pipeline35/1(d)
  bus_expand9/3(out6) -> pipeline44/1(d)
  bus_expand9/5(out4) -> pipeline48/1(d)
  bus_expand9/7(out2) -> pipeline33/1(d)
  bus_expand9/8(lsb_out1) -> pipeline34/1(d)
  bus_expand9/6(out3) -> pipeline12/1(d)
  bus_expand9/4(out5) -> pipeline46/1(d)
  bus_expand9/1(msb_out8) -> pipeline37/1(d)
  bus_expand9/2(out7) -> pipeline40/1(d)
  ss_fft/1(arm_out) -> Terminator3/1
  edge_detect13/1(out) -> ss_fft/10(trig)
  bus_expand2/2(out7) -> Gateway Out8/1
  Gateway Out8/1 -> To Workspace67/1
  Gateway Out65/1 -> To Workspace8/1
  Gateway Out9/1 -> To Workspace9/1
  bus_expand2/4(out5) -> Gateway Out9/1
  Gateway Out19/1 -> To Workspace11/1
  bus_expand2/5(out4) -> Gateway Out19/1
  Gateway Out20/1 -> To Workspace20/1
  bus_expand2/6(out3) -> Gateway Out20/1
  Gateway Out38/1 -> To Workspace38/1
  bus_expand2/7(out2) -> Gateway Out38/1
  Gateway Out39/1 -> To Workspace39/1
  bus_expand2/8(lsb_out1) -> Gateway Out39/1
  Gateway Out64/1 -> To Workspace65/1
  Register2/1 -> Delay1/1
  Logical9/1 -> edge_detect2/1(in)
  Constant6/1 -> man_sync/1(sim_1)
  Delay36/1 -> edge_detect11/1(in)
  pipeline23/1(q) -> [sys_rst]
  [man_sync] -> Logical9/2, Logical9/1
  fft_only/2(dout0) -> [pfb_d144_0]
  fft_only/3(dout1) -> [pfb_d144_1]
  fft_only/4(dvalid) -> [pfb_dv]
  fft_only/5(of) -> [pfb_of]
  Delay43/1 -> [pfb_sync]
  Constant85/1 -> fft_shift/1(sim_1)
  pipeline10/1(q) -> [wng_scale0]
  Gateway Out73/1 -> To Workspace73/1
  Gateway Out62/1 -> To Workspace64/1
  Logical20/1 -> pipeline198/1(d)
  Gateway Out42/1 -> To Workspace44/1
  Constant79/1 -> man_rst_wng/1(sim_1)
  man_rst_wng/1(in_rst) -> Logical20/2
  wng0/1(bus_out) -> bus_expand10/1(bus_in), [wng]
  bus_expand10/5(out4) -> Gateway Out51/1
  bus_expand10/2(out7) -> Gateway Out61/1
  bus_expand10/1(msb_out8) -> Gateway Out60/1
  Gateway Out67/1 -> To Workspace66/1
  Gateway Out51/1 -> To Workspace51/1
  Gateway Out61/1 -> To Workspace63/1
  [wng_scale0] -> wng0/1(scale_16_14)
  bus_expand10/8(lsb_out1) -> Gateway Out73/1
  Gateway Out63/1 -> To Workspace62/1
  bus_expand10/3(out6) -> Gateway Out42/1
  bus_expand10/6(out3) -> Gateway Out63/1
  Gateway Out45/1 -> To Workspace50/1
  bus_expand10/4(out5) -> Gateway Out45/1
  bus_expand10/7(out2) -> Gateway Out67/1
  [sys_rst] -> Logical20/1
  Gateway Out60/1 -> To Workspace61/1
  pipeline198/1(q) -> wng0/2(rst)
  scale_wng/1(in_scale) -> Gateway Out62/1, pipeline10/1(d)
  Constant80/1 -> scale_wng/1(sim_1)
  bus_convert2/1(dout) -> [d80_adc0]
  Gateway Out118/1 -> To Workspace118/1
  bus_expand8/2(lsb_out1) -> [valid80], Gateway Out118/1
  bus_addsub/1(dout) -> bus_convert2/1(din)
  [cwg80] -> bus_addsub/1(a)
  [cwg_sync] -> Concat1/1
  [cwg_dv] -> Concat1/2
  bus_expand8/1(msb_out2) -> [sync80], Gateway Out117/1
  Gateway Out117/1 -> To Workspace117/1
  Concat1/1 -> bus_addsub/3(misci)
  bus_addsub/2(misco) -> bus_convert2/2(misci)
  [wng] -> bus_addsub/2(b)
  bus_convert2/2(misco) -> bus_expand8/1(bus_in)
